simulink model "TSR_Scenario_005_cs"  (Simulink 7.6)
settings: Solver=ode5  SolverMode=SingleTasking  StartTime=0.0  StopTime=inf  FixedStep=1/100  RelTol=1e-3
PreLoadFcn:
  | check_NUTS_defined;
  | 
  | assignin('base',['model_closed_' bdroot],false);
  | 
  | addpath('../includes_TSR/includes_TSR');
  | 
  | switch version('-release')
  | case {'2010a', '2010b', '2011a'}
  | addpath('../includes_TSR/ML2010b');
  | otherwise
  | addpath('../includes_TSR/ML2011b');
  | end
InitFcn:
  | PreScanExperimentInitFcn(bdroot)
StopFcn:
  | PreScanExperimentStopFcn(bdroot)
undefined symbols (not defined in the file): DiscIntNeverNeededParam, InitImageOutputDir, x_ini_VRML, y_ini_VRML, z_ini_VRML
libraries: PreScanSimulinkLibrary x97, simulink x59, PreScanUsefulBlocks x33, simulink_extras x30, simulink_need_slupdate x1
(part 3 of 26)

== system TSR_Scenario_005_cs/FullCurtainSidedTrailer_1 ==
  SELF_FullCurtainSidedTrailer_1 : Inport  Tag=SELFInport
  Dynamics_Empty : Subsystem  Tag=Dynamics_Empty
      mask Display: port_label('output', 1, 'State');text(0,0,' Empty\n')
  SELF_Demux : Subsystem  Tag=DemuxSELF
      settings: DeleteFcn=PreScanBlockDeleteFcn;
      mask Type: PreScan Demux Block:
      mask Display:
      | port_label('output',1,'x [m]')
      | port_label('output',2,'y [m]')
      | port_label('output',3,'z [m]','texmode','on')
      | port_label('output',4,'Rotx [deg]','texmode','on')
      | port_label('output',5,'Roty [deg]','texmode','on')
      | port_label('output',6,'Rotz [deg]','texmode','on')
      | port_label('output',7,'GPS lat [deg]','texmode','on')
      | port_label('output',8,'GPS long [deg]','texmode','on')
      | port_label('output',9,'GPS alt [m]','texmode','on')
      | port_label('output',10,'Velocity [m/s]')
      | port_label('output',11,'Heading [deg]')
      | port_label('output',12,'Yaw rate [deg/s]')
  Terminator_{1..12} (12 alike) : Terminator
  STATE_FullCurtainSidedTrailer_1 : Outport  Tag=StateOutport
  wires:
  SELF_FullCurtainSidedTrailer_1/1 -> SELF_Demux/1(SELF)
  Dynamics_Empty/1(State) -> STATE_FullCurtainSidedTrailer_1/1
  SELF_Demux/1(x [m]) -> Terminator_1/1
  SELF_Demux/2(y [m]) -> Terminator_2/1
  SELF_Demux/3(z [m]) -> Terminator_3/1
  SELF_Demux/4(rotx [deg]) -> Terminator_4/1
  SELF_Demux/5(roty [deg]) -> Terminator_5/1
  SELF_Demux/6(rotz [deg]) -> Terminator_6/1
  SELF_Demux/7(GPS lat [deg]) -> Terminator_7/1
  SELF_Demux/8(GPS long [deg]) -> Terminator_8/1
  SELF_Demux/9(GPS alt [m]) -> Terminator_9/1
  SELF_Demux/10(velocity [m/s]) -> Terminator_10/1
  SELF_Demux/11(heading [deg]) -> Terminator_11/1
  SELF_Demux/12(yaw rate [deg/s]) -> Terminator_12/1

== system TSR_Scenario_005_cs/GetSensors ==
  dummy : Inport
  GetSensors : M-S-Function  FunctionName=GetSensorsBlock  Parameters=TimeStep NrOfSensors
  tag scope: SimCoreSensors=global
  wires:
  GetSensors/1 -> [SimCoreSensors]
  dummy/1 -> GetSensors/1

== system TSR_Scenario_005_cs/Honda_Pan_European_1 ==
  SELF_Honda_Pan_European_1 : Inport  Tag=SELFInport
  SELF_Demux : Subsystem  Tag=DemuxSELF
      settings: DeleteFcn=PreScanBlockDeleteFcn;
      mask Type: PreScan Demux Block:
      mask Display:
      | port_label('output',1,'x [m]')
      | port_label('output',2,'y [m]')
      | port_label('output',3,'z [m]','texmode','on')
      | port_label('output',4,'Rotx [deg]','texmode','on')
      | port_label('output',5,'Roty [deg]','texmode','on')
      | port_label('output',6,'Rotz [deg]','texmode','on')
      | port_label('output',7,'GPS lat [deg]','texmode','on')
      | port_label('output',8,'GPS long [deg]','texmode','on')
      | port_label('output',9,'GPS alt [m]','texmode','on')
      | port_label('output',10,'Velocity [m/s]')
      | port_label('output',11,'Heading [deg]')
      | port_label('output',12,'Yaw rate [deg/s]')
  Terminator_{1..12} (12 alike) : Terminator
  Trajectories : Subsystem  Tag=Trajectories
      settings: DeleteFcn=PreScanBlockDeleteFcn;
      mask Type: PreScan Source Block: Trajectories
      mask Description:
      | The Trajectory block contains the trajectories defined in the PreScan Experiment Editor. The output of the trajectory block is the (vehicle) State.
      | 
      | For more information, please click Help.
      mask Help: eval('openHelp(312)')
      mask Display: image(imread('Trajectory.png'));
  STATE_Honda_Pan_European_1 : Outport  Tag=StateOutport
  wires:
  SELF_Honda_Pan_European_1/1 -> SELF_Demux/1(SELF)
  Trajectories/1(State) -> STATE_Honda_Pan_European_1/1
  SELF_Demux/1(x [m]) -> Terminator_1/1
  SELF_Demux/2(y [m]) -> Terminator_2/1
  SELF_Demux/3(z [m]) -> Terminator_3/1
  SELF_Demux/4(rotx [deg]) -> Terminator_4/1
  SELF_Demux/5(roty [deg]) -> Terminator_5/1
  SELF_Demux/6(rotz [deg]) -> Terminator_6/1
  SELF_Demux/7(GPS lat [deg]) -> Terminator_7/1
  SELF_Demux/8(GPS long [deg]) -> Terminator_8/1
  SELF_Demux/9(GPS alt [m]) -> Terminator_9/1
  SELF_Demux/10(velocity [m/s]) -> Terminator_10/1
  SELF_Demux/11(heading [deg]) -> Terminator_11/1
  SELF_Demux/12(yaw rate [deg/s]) -> Terminator_12/1

== system TSR_Scenario_005_cs/Lightpost_1 ==
  note: Resources
      |
      | 1: LightMap\HeadLight LB and HB.png: HeadLight LB and HB
      | 2: LightMap\HeadLight LB.png: HeadLight LB
      | 3: LightMap\GeneralLight.png: GeneralLight
      | 4: LightMap\BrakeLight.png: BrakeLight
  note: Resources
  LightPostLight_Col : Constant  Tag=VisuLightConst  Value=[255 210 89]
  LightPostLight_ID : Constant  Tag=VisuLightConst  Value=[0]
  LightPostLight_Ori : Constant  Tag=VisuLightConst  Value=[0 0 0]
  LightPostLight_Pos : Constant  Tag=VisuLightConst  Value=[3.2599999904632568 -1.7702632248983718E-05 9.9388999938964844]
  LightPostLight_mscd : Constant  Tag=VisuLightConst  Value=[398.33]
  VISULightPostLight_Mux : Subsystem  Tag=MuxLights
      settings: DeleteFcn=PreScanBlockDeleteFcn;
      mask Type: PreScan Mux Block:
  VISULightPostLight_Lightpost_1 : Outport  Tag=VisuLightPostLightOutport  SampleTime=1/100
  wires:
  VISULightPostLight_Mux/1(Lights) -> VISULightPostLight_Lightpost_1/1
  LightPostLight_mscd/1 -> VISULightPostLight_Mux/1(MSCD [Cd])
  LightPostLight_Pos/1 -> VISULightPostLight_Mux/2(Position (xyz) [m])
  LightPostLight_Ori/1 -> VISULightPostLight_Mux/3(Orientation (RPY) [deg])
  LightPostLight_Col/1 -> VISULightPostLight_Mux/4(Color [-])
  LightPostLight_ID/1 -> VISULightPostLight_Mux/5(TextureResourceID [-])

== system TSR_Scenario_005_cs/Lightpost_10 ==

== system TSR_Scenario_005_cs/Lightpost_11 ==
  note: Resources
      |
      | 1: LightMap\HeadLight LB and HB.png: HeadLight LB and HB
      | 2: LightMap\HeadLight LB.png: HeadLight LB
      | 3: LightMap\GeneralLight.png: GeneralLight
      | 4: LightMap\BrakeLight.png: BrakeLight
  note: Resources
  LightPostLight_Col : Constant  Tag=VisuLightConst  Value=[255 210 89]
  LightPostLight_ID : Constant  Tag=VisuLightConst  Value=[0]
  LightPostLight_Ori : Constant  Tag=VisuLightConst  Value=[0 0 0]
  LightPostLight_Pos : Constant  Tag=VisuLightConst  Value=[3.2599999904632568 -1.7702632248983718E-05 9.9388999938964844]
  LightPostLight_mscd : Constant  Tag=VisuLightConst  Value=[398.33]
  VISULightPostLight_Mux : Subsystem  Tag=MuxLights
      settings: DeleteFcn=PreScanBlockDeleteFcn;
      mask Type: PreScan Mux Block:
  VISULightPostLight_Lightpost_11 : Outport  Tag=VisuLightPostLightOutport  SampleTime=1/100
  wires:
  VISULightPostLight_Mux/1(Lights) -> VISULightPostLight_Lightpost_11/1
  LightPostLight_mscd/1 -> VISULightPostLight_Mux/1(MSCD [Cd])
  LightPostLight_Pos/1 -> VISULightPostLight_Mux/2(Position (xyz) [m])
  LightPostLight_Ori/1 -> VISULightPostLight_Mux/3(Orientation (RPY) [deg])
  LightPostLight_Col/1 -> VISULightPostLight_Mux/4(Color [-])
  LightPostLight_ID/1 -> VISULightPostLight_Mux/5(TextureResourceID [-])

== system TSR_Scenario_005_cs/Lightpost_12 ==
  note: Resources
      |
      | 1: LightMap\HeadLight LB and HB.png: HeadLight LB and HB
      | 2: LightMap\HeadLight LB.png: HeadLight LB
      | 3: LightMap\GeneralLight.png: GeneralLight
      | 4: LightMap\BrakeLight.png: BrakeLight
  note: Resources
  LightPostLight_Col : Constant  Tag=VisuLightConst  Value=[255 210 89]
  LightPostLight_ID : Constant  Tag=VisuLightConst  Value=[0]
  LightPostLight_Ori : Constant  Tag=VisuLightConst  Value=[0 0 0]
  LightPostLight_Pos : Constant  Tag=VisuLightConst  Value=[3.2599999904632568 -1.7702632248983718E-05 9.9388999938964844]
  LightPostLight_mscd : Constant  Tag=VisuLightConst  Value=[398.33]
  VISULightPostLight_Mux : Subsystem  Tag=MuxLights
      settings: DeleteFcn=PreScanBlockDeleteFcn;
      mask Type: PreScan Mux Block:
  VISULightPostLight_Lightpost_12 : Outport  Tag=VisuLightPostLightOutport  SampleTime=1/100
  wires:
  VISULightPostLight_Mux/1(Lights) -> VISULightPostLight_Lightpost_12/1
  LightPostLight_mscd/1 -> VISULightPostLight_Mux/1(MSCD [Cd])
  LightPostLight_Pos/1 -> VISULightPostLight_Mux/2(Position (xyz) [m])
  LightPostLight_Ori/1 -> VISULightPostLight_Mux/3(Orientation (RPY) [deg])
  LightPostLight_Col/1 -> VISULightPostLight_Mux/4(Color [-])
  LightPostLight_ID/1 -> VISULightPostLight_Mux/5(TextureResourceID [-])

== system TSR_Scenario_005_cs/Lightpost_13 ==

== system TSR_Scenario_005_cs/Lightpost_14 ==

== system TSR_Scenario_005_cs/Lightpost_15 ==

== system TSR_Scenario_005_cs/Lightpost_16 ==

== system TSR_Scenario_005_cs/Lightpost_17 ==

== system TSR_Scenario_005_cs/Lightpost_18 ==

== system TSR_Scenario_005_cs/Lightpost_19 ==

== system TSR_Scenario_005_cs/Lightpost_2 ==
  note: Resources
      |
      | 1: LightMap\HeadLight LB and HB.png: HeadLight LB and HB
      | 2: LightMap\HeadLight LB.png: HeadLight LB
      | 3: LightMap\GeneralLight.png: GeneralLight
      | 4: LightMap\BrakeLight.png: BrakeLight
  note: Resources
  LightPostLight_Col : Constant  Tag=VisuLightConst  Value=[255 210 89]
  LightPostLight_ID : Constant  Tag=VisuLightConst  Value=[0]
  LightPostLight_Ori : Constant  Tag=VisuLightConst  Value=[0 0 0]
  LightPostLight_Pos : Constant  Tag=VisuLightConst  Value=[3.2599999904632568 -1.7702632248983718E-05 9.9388999938964844]
  LightPostLight_mscd : Constant  Tag=VisuLightConst  Value=[398.33]
  VISULightPostLight_Mux : Subsystem  Tag=MuxLights
      settings: DeleteFcn=PreScanBlockDeleteFcn;
      mask Type: PreScan Mux Block:
  VISULightPostLight_Lightpost_2 : Outport  Tag=VisuLightPostLightOutport  SampleTime=1/100
  wires:
  VISULightPostLight_Mux/1(Lights) -> VISULightPostLight_Lightpost_2/1
  LightPostLight_mscd/1 -> VISULightPostLight_Mux/1(MSCD [Cd])
  LightPostLight_Pos/1 -> VISULightPostLight_Mux/2(Position (xyz) [m])
  LightPostLight_Ori/1 -> VISULightPostLight_Mux/3(Orientation (RPY) [deg])
  LightPostLight_Col/1 -> VISULightPostLight_Mux/4(Color [-])
  LightPostLight_ID/1 -> VISULightPostLight_Mux/5(TextureResourceID [-])

== system TSR_Scenario_005_cs/Lightpost_20 ==

== system TSR_Scenario_005_cs/Lightpost_21 ==

== system TSR_Scenario_005_cs/Lightpost_22 ==

== system TSR_Scenario_005_cs/Lightpost_23 ==

== system TSR_Scenario_005_cs/Lightpost_24 ==

== system TSR_Scenario_005_cs/Lightpost_25 ==
  note: Resources
      |
      | 1: LightMap\HeadLight LB and HB.png: HeadLight LB and HB
      | 2: LightMap\HeadLight LB.png: HeadLight LB
      | 3: LightMap\GeneralLight.png: GeneralLight
      | 4: LightMap\BrakeLight.png: BrakeLight
  note: Resources
  LightPostLight_Col : Constant  Tag=VisuLightConst  Value=[255 210 89]
  LightPostLight_ID : Constant  Tag=VisuLightConst  Value=[0]
  LightPostLight_Ori : Constant  Tag=VisuLightConst  Value=[0 0 0]
  LightPostLight_Pos : Constant  Tag=VisuLightConst  Value=[3.2599999904632568 -1.7702632248983718E-05 9.9388999938964844]
  LightPostLight_mscd : Constant  Tag=VisuLightConst  Value=[398.33]
  VISULightPostLight_Mux : Subsystem  Tag=MuxLights
      settings: DeleteFcn=PreScanBlockDeleteFcn;
      mask Type: PreScan Mux Block:
  VISULightPostLight_Lightpost_25 : Outport  Tag=VisuLightPostLightOutport  SampleTime=1/100
  wires:
  VISULightPostLight_Mux/1(Lights) -> VISULightPostLight_Lightpost_25/1
  LightPostLight_mscd/1 -> VISULightPostLight_Mux/1(MSCD [Cd])
  LightPostLight_Pos/1 -> VISULightPostLight_Mux/2(Position (xyz) [m])
  LightPostLight_Ori/1 -> VISULightPostLight_Mux/3(Orientation (RPY) [deg])
  LightPostLight_Col/1 -> VISULightPostLight_Mux/4(Color [-])
  LightPostLight_ID/1 -> VISULightPostLight_Mux/5(TextureResourceID [-])

== system TSR_Scenario_005_cs/Lightpost_26 ==

== system TSR_Scenario_005_cs/Lightpost_27 ==
  note: Resources
      |
      | 1: LightMap\HeadLight LB and HB.png: HeadLight LB and HB
      | 2: LightMap\HeadLight LB.png: HeadLight LB
      | 3: LightMap\GeneralLight.png: GeneralLight
      | 4: LightMap\BrakeLight.png: BrakeLight
  note: Resources
  LightPostLight_Col : Constant  Tag=VisuLightConst  Value=[255 210 89]
  LightPostLight_ID : Constant  Tag=VisuLightConst  Value=[0]
  LightPostLight_Ori : Constant  Tag=VisuLightConst  Value=[0 0 0]
  LightPostLight_Pos : Constant  Tag=VisuLightConst  Value=[3.2599999904632568 -1.7702632248983718E-05 9.9388999938964844]
  LightPostLight_mscd : Constant  Tag=VisuLightConst  Value=[398.33]
  VISULightPostLight_Mux : Subsystem  Tag=MuxLights
      settings: DeleteFcn=PreScanBlockDeleteFcn;
      mask Type: PreScan Mux Block:
  VISULightPostLight_Lightpost_27 : Outport  Tag=VisuLightPostLightOutport  SampleTime=1/100
  wires:
  VISULightPostLight_Mux/1(Lights) -> VISULightPostLight_Lightpost_27/1
  LightPostLight_mscd/1 -> VISULightPostLight_Mux/1(MSCD [Cd])
  LightPostLight_Pos/1 -> VISULightPostLight_Mux/2(Position (xyz) [m])
  LightPostLight_Ori/1 -> VISULightPostLight_Mux/3(Orientation (RPY) [deg])
  LightPostLight_Col/1 -> VISULightPostLight_Mux/4(Color [-])
  LightPostLight_ID/1 -> VISULightPostLight_Mux/5(TextureResourceID [-])

== system TSR_Scenario_005_cs/Lightpost_28 ==

== system TSR_Scenario_005_cs/Lightpost_29 ==

== system TSR_Scenario_005_cs/Lightpost_3 ==
  note: Resources
      |
      | 1: LightMap\HeadLight LB and HB.png: HeadLight LB and HB
      | 2: LightMap\HeadLight LB.png: HeadLight LB
      | 3: LightMap\GeneralLight.png: GeneralLight
      | 4: LightMap\BrakeLight.png: BrakeLight
  note: Resources
  LightPostLight_Col : Constant  Tag=VisuLightConst  Value=[255 210 89]
  LightPostLight_ID : Constant  Tag=VisuLightConst  Value=[0]
  LightPostLight_Ori : Constant  Tag=VisuLightConst  Value=[0 0 0]
  LightPostLight_Pos : Constant  Tag=VisuLightConst  Value=[3.2599999904632568 -1.7702632248983718E-05 9.9388999938964844]
  LightPostLight_mscd : Constant  Tag=VisuLightConst  Value=[398.33]
  VISULightPostLight_Mux : Subsystem  Tag=MuxLights
      settings: DeleteFcn=PreScanBlockDeleteFcn;
      mask Type: PreScan Mux Block:
  VISULightPostLight_Lightpost_3 : Outport  Tag=VisuLightPostLightOutport  SampleTime=1/100
  wires:
  VISULightPostLight_Mux/1(Lights) -> VISULightPostLight_Lightpost_3/1
  LightPostLight_mscd/1 -> VISULightPostLight_Mux/1(MSCD [Cd])
  LightPostLight_Pos/1 -> VISULightPostLight_Mux/2(Position (xyz) [m])
  LightPostLight_Ori/1 -> VISULightPostLight_Mux/3(Orientation (RPY) [deg])
  LightPostLight_Col/1 -> VISULightPostLight_Mux/4(Color [-])
  LightPostLight_ID/1 -> VISULightPostLight_Mux/5(TextureResourceID [-])

== system TSR_Scenario_005_cs/Lightpost_30 ==

== system TSR_Scenario_005_cs/Lightpost_31 ==

== system TSR_Scenario_005_cs/Lightpost_32 ==

== system TSR_Scenario_005_cs/Lightpost_33 ==

== system TSR_Scenario_005_cs/Lightpost_34 ==
  note: Resources
      |
      | 1: LightMap\HeadLight LB and HB.png: HeadLight LB and HB
      | 2: LightMap\HeadLight LB.png: HeadLight LB
      | 3: LightMap\GeneralLight.png: GeneralLight
      | 4: LightMap\BrakeLight.png: BrakeLight
  note: Resources
  LightPostLight_Col : Constant  Tag=VisuLightConst  Value=[255 210 89]
  LightPostLight_ID : Constant  Tag=VisuLightConst  Value=[0]
  LightPostLight_Ori : Constant  Tag=VisuLightConst  Value=[0 0 0]
  LightPostLight_Pos : Constant  Tag=VisuLightConst  Value=[3.2599999904632568 -1.7702632248983718E-05 9.9388999938964844]
  LightPostLight_mscd : Constant  Tag=VisuLightConst  Value=[398.33]
  VISULightPostLight_Mux : Subsystem  Tag=MuxLights
      settings: DeleteFcn=PreScanBlockDeleteFcn;
      mask Type: PreScan Mux Block:
  VISULightPostLight_Lightpost_34 : Outport  Tag=VisuLightPostLightOutport  SampleTime=1/100
  wires:
  VISULightPostLight_Mux/1(Lights) -> VISULightPostLight_Lightpost_34/1
  LightPostLight_mscd/1 -> VISULightPostLight_Mux/1(MSCD [Cd])
  LightPostLight_Pos/1 -> VISULightPostLight_Mux/2(Position (xyz) [m])
  LightPostLight_Ori/1 -> VISULightPostLight_Mux/3(Orientation (RPY) [deg])
  LightPostLight_Col/1 -> VISULightPostLight_Mux/4(Color [-])
  LightPostLight_ID/1 -> VISULightPostLight_Mux/5(TextureResourceID [-])

== system TSR_Scenario_005_cs/Lightpost_35 ==

== system TSR_Scenario_005_cs/Lightpost_36 ==
  note: Resources
      |
      | 1: LightMap\HeadLight LB and HB.png: HeadLight LB and HB
      | 2: LightMap\HeadLight LB.png: HeadLight LB
      | 3: LightMap\GeneralLight.png: GeneralLight
      | 4: LightMap\BrakeLight.png: BrakeLight
  note: Resources
  LightPostLight_Col : Constant  Tag=VisuLightConst  Value=[255 210 89]
  LightPostLight_ID : Constant  Tag=VisuLightConst  Value=[0]
  LightPostLight_Ori : Constant  Tag=VisuLightConst  Value=[0 0 0]
  LightPostLight_Pos : Constant  Tag=VisuLightConst  Value=[3.2599999904632568 -1.7702632248983718E-05 9.9388999938964844]
  LightPostLight_mscd : Constant  Tag=VisuLightConst  Value=[398.33]
  VISULightPostLight_Mux : Subsystem  Tag=MuxLights
      settings: DeleteFcn=PreScanBlockDeleteFcn;
      mask Type: PreScan Mux Block:
  VISULightPostLight_Lightpost_36 : Outport  Tag=VisuLightPostLightOutport  SampleTime=1/100
  wires:
  VISULightPostLight_Mux/1(Lights) -> VISULightPostLight_Lightpost_36/1
  LightPostLight_mscd/1 -> VISULightPostLight_Mux/1(MSCD [Cd])
  LightPostLight_Pos/1 -> VISULightPostLight_Mux/2(Position (xyz) [m])
  LightPostLight_Ori/1 -> VISULightPostLight_Mux/3(Orientation (RPY) [deg])
  LightPostLight_Col/1 -> VISULightPostLight_Mux/4(Color [-])
  LightPostLight_ID/1 -> VISULightPostLight_Mux/5(TextureResourceID [-])

== system TSR_Scenario_005_cs/Lightpost_37 ==

== system TSR_Scenario_005_cs/Lightpost_38 ==
  note: Resources
      |
      | 1: LightMap\HeadLight LB and HB.png: HeadLight LB and HB
      | 2: LightMap\HeadLight LB.png: HeadLight LB
      | 3: LightMap\GeneralLight.png: GeneralLight
      | 4: LightMap\BrakeLight.png: BrakeLight
  note: Resources
  LightPostLight_Col : Constant  Tag=VisuLightConst  Value=[255 210 89]
  LightPostLight_ID : Constant  Tag=VisuLightConst  Value=[0]
  LightPostLight_Ori : Constant  Tag=VisuLightConst  Value=[0 0 0]
  LightPostLight_Pos : Constant  Tag=VisuLightConst  Value=[3.2599999904632568 -1.7702632248983718E-05 9.9388999938964844]
  LightPostLight_mscd : Constant  Tag=VisuLightConst  Value=[398.33]
  VISULightPostLight_Mux : Subsystem  Tag=MuxLights
      settings: DeleteFcn=PreScanBlockDeleteFcn;
      mask Type: PreScan Mux Block:
  VISULightPostLight_Lightpost_38 : Outport  Tag=VisuLightPostLightOutport  SampleTime=1/100
  wires:
  VISULightPostLight_Mux/1(Lights) -> VISULightPostLight_Lightpost_38/1
  LightPostLight_mscd/1 -> VISULightPostLight_Mux/1(MSCD [Cd])
  LightPostLight_Pos/1 -> VISULightPostLight_Mux/2(Position (xyz) [m])
  LightPostLight_Ori/1 -> VISULightPostLight_Mux/3(Orientation (RPY) [deg])
  LightPostLight_Col/1 -> VISULightPostLight_Mux/4(Color [-])
  LightPostLight_ID/1 -> VISULightPostLight_Mux/5(TextureResourceID [-])

== system TSR_Scenario_005_cs/Lightpost_39 ==

== system TSR_Scenario_005_cs/Lightpost_4 ==
  note: Resources
      |
      | 1: LightMap\HeadLight LB and HB.png: HeadLight LB and HB
      | 2: LightMap\HeadLight LB.png: HeadLight LB
      | 3: LightMap\GeneralLight.png: GeneralLight
      | 4: LightMap\BrakeLight.png: BrakeLight
  note: Resources
  LightPostLight_Col : Constant  Tag=VisuLightConst  Value=[255 210 89]
  LightPostLight_ID : Constant  Tag=VisuLightConst  Value=[0]
  LightPostLight_Ori : Constant  Tag=VisuLightConst  Value=[0 0 0]
  LightPostLight_Pos : Constant  Tag=VisuLightConst  Value=[3.2599999904632568 -1.7702632248983718E-05 9.9388999938964844]
  LightPostLight_mscd : Constant  Tag=VisuLightConst  Value=[398.33]
  VISULightPostLight_Mux : Subsystem  Tag=MuxLights
      settings: DeleteFcn=PreScanBlockDeleteFcn;
      mask Type: PreScan Mux Block:
  VISULightPostLight_Lightpost_4 : Outport  Tag=VisuLightPostLightOutport  SampleTime=1/100
  wires:
  VISULightPostLight_Mux/1(Lights) -> VISULightPostLight_Lightpost_4/1
  LightPostLight_mscd/1 -> VISULightPostLight_Mux/1(MSCD [Cd])
  LightPostLight_Pos/1 -> VISULightPostLight_Mux/2(Position (xyz) [m])
  LightPostLight_Ori/1 -> VISULightPostLight_Mux/3(Orientation (RPY) [deg])
  LightPostLight_Col/1 -> VISULightPostLight_Mux/4(Color [-])
  LightPostLight_ID/1 -> VISULightPostLight_Mux/5(TextureResourceID [-])

== system TSR_Scenario_005_cs/Lightpost_40 ==
  note: Resources
      |
      | 1: LightMap\HeadLight LB and HB.png: HeadLight LB and HB
      | 2: LightMap\HeadLight LB.png: HeadLight LB
      | 3: LightMap\GeneralLight.png: GeneralLight
      | 4: LightMap\BrakeLight.png: BrakeLight
  note: Resources
  LightPostLight_Col : Constant  Tag=VisuLightConst  Value=[255 210 89]
  LightPostLight_ID : Constant  Tag=VisuLightConst  Value=[0]
  LightPostLight_Ori : Constant  Tag=VisuLightConst  Value=[0 0 0]
  LightPostLight_Pos : Constant  Tag=VisuLightConst  Value=[3.2599999904632568 -1.7702632248983718E-05 9.9388999938964844]
  LightPostLight_mscd : Constant  Tag=VisuLightConst  Value=[398.33]
  VISULightPostLight_Mux : Subsystem  Tag=MuxLights
      settings: DeleteFcn=PreScanBlockDeleteFcn;
      mask Type: PreScan Mux Block:
  VISULightPostLight_Lightpost_40 : Outport  Tag=VisuLightPostLightOutport  SampleTime=1/100
  wires:
  VISULightPostLight_Mux/1(Lights) -> VISULightPostLight_Lightpost_40/1
  LightPostLight_mscd/1 -> VISULightPostLight_Mux/1(MSCD [Cd])
  LightPostLight_Pos/1 -> VISULightPostLight_Mux/2(Position (xyz) [m])
  LightPostLight_Ori/1 -> VISULightPostLight_Mux/3(Orientation (RPY) [deg])
  LightPostLight_Col/1 -> VISULightPostLight_Mux/4(Color [-])
  LightPostLight_ID/1 -> VISULightPostLight_Mux/5(TextureResourceID [-])

== system TSR_Scenario_005_cs/Lightpost_41 ==

== system TSR_Scenario_005_cs/Lightpost_42 ==
  note: Resources
      |
      | 1: LightMap\HeadLight LB and HB.png: HeadLight LB and HB
      | 2: LightMap\HeadLight LB.png: HeadLight LB
      | 3: LightMap\GeneralLight.png: GeneralLight
      | 4: LightMap\BrakeLight.png: BrakeLight
  note: Resources
  LightPostLight_Col : Constant  Tag=VisuLightConst  Value=[255 210 89]
  LightPostLight_ID : Constant  Tag=VisuLightConst  Value=[0]
  LightPostLight_Ori : Constant  Tag=VisuLightConst  Value=[0 0 0]
  LightPostLight_Pos : Constant  Tag=VisuLightConst  Value=[3.2599999904632568 -1.7702632248983718E-05 9.9388999938964844]
  LightPostLight_mscd : Constant  Tag=VisuLightConst  Value=[398.33]
  VISULightPostLight_Mux : Subsystem  Tag=MuxLights
      settings: DeleteFcn=PreScanBlockDeleteFcn;
      mask Type: PreScan Mux Block:
  VISULightPostLight_Lightpost_42 : Outport  Tag=VisuLightPostLightOutport  SampleTime=1/100
  wires:
  VISULightPostLight_Mux/1(Lights) -> VISULightPostLight_Lightpost_42/1
  LightPostLight_mscd/1 -> VISULightPostLight_Mux/1(MSCD [Cd])
  LightPostLight_Pos/1 -> VISULightPostLight_Mux/2(Position (xyz) [m])
  LightPostLight_Ori/1 -> VISULightPostLight_Mux/3(Orientation (RPY) [deg])
  LightPostLight_Col/1 -> VISULightPostLight_Mux/4(Color [-])
  LightPostLight_ID/1 -> VISULightPostLight_Mux/5(TextureResourceID [-])

== system TSR_Scenario_005_cs/Lightpost_43 ==

== system TSR_Scenario_005_cs/Lightpost_44 ==
  note: Resources
      |
      | 1: LightMap\HeadLight LB and HB.png: HeadLight LB and HB
      | 2: LightMap\HeadLight LB.png: HeadLight LB
      | 3: LightMap\GeneralLight.png: GeneralLight
      | 4: LightMap\BrakeLight.png: BrakeLight
  note: Resources
  LightPostLight_Col : Constant  Tag=VisuLightConst  Value=[255 210 89]
  LightPostLight_ID : Constant  Tag=VisuLightConst  Value=[0]
  LightPostLight_Ori : Constant  Tag=VisuLightConst  Value=[0 0 0]
  LightPostLight_Pos : Constant  Tag=VisuLightConst  Value=[3.2599999904632568 -1.7702632248983718E-05 9.9388999938964844]
  LightPostLight_mscd : Constant  Tag=VisuLightConst  Value=[398.33]
  VISULightPostLight_Mux : Subsystem  Tag=MuxLights
      settings: DeleteFcn=PreScanBlockDeleteFcn;
      mask Type: PreScan Mux Block:
  VISULightPostLight_Lightpost_44 : Outport  Tag=VisuLightPostLightOutport  SampleTime=1/100
  wires:
  VISULightPostLight_Mux/1(Lights) -> VISULightPostLight_Lightpost_44/1
  LightPostLight_mscd/1 -> VISULightPostLight_Mux/1(MSCD [Cd])
  LightPostLight_Pos/1 -> VISULightPostLight_Mux/2(Position (xyz) [m])
  LightPostLight_Ori/1 -> VISULightPostLight_Mux/3(Orientation (RPY) [deg])
  LightPostLight_Col/1 -> VISULightPostLight_Mux/4(Color [-])
  LightPostLight_ID/1 -> VISULightPostLight_Mux/5(TextureResourceID [-])

== system TSR_Scenario_005_cs/Lightpost_45 ==

== system TSR_Scenario_005_cs/Lightpost_46 ==

== system TSR_Scenario_005_cs/Lightpost_47 ==

== system TSR_Scenario_005_cs/Lightpost_48 ==

== system TSR_Scenario_005_cs/Lightpost_49 ==

== system TSR_Scenario_005_cs/Lightpost_5 ==
  note: Resources
      |
      | 1: LightMap\HeadLight LB and HB.png: HeadLight LB and HB
      | 2: LightMap\HeadLight LB.png: HeadLight LB
      | 3: LightMap\GeneralLight.png: GeneralLight
      | 4: LightMap\BrakeLight.png: BrakeLight
  note: Resources
  LightPostLight_Col : Constant  Tag=VisuLightConst  Value=[255 210 89]
  LightPostLight_ID : Constant  Tag=VisuLightConst  Value=[0]
  LightPostLight_Ori : Constant  Tag=VisuLightConst  Value=[0 0 0]
  LightPostLight_Pos : Constant  Tag=VisuLightConst  Value=[3.2599999904632568 -1.7702632248983718E-05 9.9388999938964844]
  LightPostLight_mscd : Constant  Tag=VisuLightConst  Value=[398.33]
  VISULightPostLight_Mux : Subsystem  Tag=MuxLights
      settings: DeleteFcn=PreScanBlockDeleteFcn;
      mask Type: PreScan Mux Block:
  VISULightPostLight_Lightpost_5 : Outport  Tag=VisuLightPostLightOutport  SampleTime=1/100
  wires:
  VISULightPostLight_Mux/1(Lights) -> VISULightPostLight_Lightpost_5/1
  LightPostLight_mscd/1 -> VISULightPostLight_Mux/1(MSCD [Cd])
  LightPostLight_Pos/1 -> VISULightPostLight_Mux/2(Position (xyz) [m])
  LightPostLight_Ori/1 -> VISULightPostLight_Mux/3(Orientation (RPY) [deg])
  LightPostLight_Col/1 -> VISULightPostLight_Mux/4(Color [-])
  LightPostLight_ID/1 -> VISULightPostLight_Mux/5(TextureResourceID [-])

== system TSR_Scenario_005_cs/Lightpost_50 ==

== system TSR_Scenario_005_cs/Lightpost_51 ==

== system TSR_Scenario_005_cs/Lightpost_52 ==

== system TSR_Scenario_005_cs/Lightpost_53 ==

== system TSR_Scenario_005_cs/Lightpost_54 ==
  note: Resources
      |
      | 1: LightMap\HeadLight LB and HB.png: HeadLight LB and HB
      | 2: LightMap\HeadLight LB.png: HeadLight LB
      | 3: LightMap\GeneralLight.png: GeneralLight
      | 4: LightMap\BrakeLight.png: BrakeLight
  note: Resources
  LightPostLight_Col : Constant  Tag=VisuLightConst  Value=[255 210 89]
  LightPostLight_ID : Constant  Tag=VisuLightConst  Value=[0]
  LightPostLight_Ori : Constant  Tag=VisuLightConst  Value=[0 0 0]
  LightPostLight_Pos : Constant  Tag=VisuLightConst  Value=[3.2599999904632568 -1.7702632248983718E-05 9.9388999938964844]
  LightPostLight_mscd : Constant  Tag=VisuLightConst  Value=[398.33]
  VISULightPostLight_Mux : Subsystem  Tag=MuxLights
      settings: DeleteFcn=PreScanBlockDeleteFcn;
      mask Type: PreScan Mux Block:
  VISULightPostLight_Lightpost_54 : Outport  Tag=VisuLightPostLightOutport  SampleTime=1/100
  wires:
  VISULightPostLight_Mux/1(Lights) -> VISULightPostLight_Lightpost_54/1
  LightPostLight_mscd/1 -> VISULightPostLight_Mux/1(MSCD [Cd])
  LightPostLight_Pos/1 -> VISULightPostLight_Mux/2(Position (xyz) [m])
  LightPostLight_Ori/1 -> VISULightPostLight_Mux/3(Orientation (RPY) [deg])
  LightPostLight_Col/1 -> VISULightPostLight_Mux/4(Color [-])
  LightPostLight_ID/1 -> VISULightPostLight_Mux/5(TextureResourceID [-])

== system TSR_Scenario_005_cs/Lightpost_6 ==

== system TSR_Scenario_005_cs/Lightpost_7 ==

== system TSR_Scenario_005_cs/Lightpost_8 ==

== system TSR_Scenario_005_cs/Lightpost_9 ==

== system TSR_Scenario_005_cs/Male_RegularAni_1 ==
  SELF_Male_RegularAni_1 : Inport  Tag=SELFInport
  SELF_Demux : Subsystem  Tag=DemuxSELF
      settings: DeleteFcn=PreScanBlockDeleteFcn;
      mask Type: PreScan Demux Block:
      mask Display:
      | port_label('output',1,'x [m]')
      | port_label('output',2,'y [m]')
      | port_label('output',3,'z [m]','texmode','on')
      | port_label('output',4,'Rotx [deg]','texmode','on')
      | port_label('output',5,'Roty [deg]','texmode','on')
      | port_label('output',6,'Rotz [deg]','texmode','on')
      | port_label('output',7,'GPS lat [deg]','texmode','on')
      | port_label('output',8,'GPS long [deg]','texmode','on')
      | port_label('output',9,'GPS alt [m]','texmode','on')
      | port_label('output',10,'Velocity [m/s]')
      | port_label('output',11,'Heading [deg]')
      | port_label('output',12,'Yaw rate [deg/s]')
  Terminator_{1..12} (12 alike) : Terminator
  Trajectories : Subsystem  Tag=Trajectories
      settings: DeleteFcn=PreScanBlockDeleteFcn;
      mask Type: PreScan Source Block: Trajectories
      mask Description:
      | The Trajectory block contains the trajectories defined in the PreScan Experiment Editor. The output of the trajectory block is the (vehicle) State.
      | 
      | For more information, please click Help.
      mask Help: eval('openHelp(312)')
      mask Display: image(imread('Trajectory.png'));
  STATE_Male_RegularAni_1 : Outport  Tag=StateOutport
  wires:
  SELF_Male_RegularAni_1/1 -> SELF_Demux/1(SELF)
  Trajectories/1(State) -> STATE_Male_RegularAni_1/1
  SELF_Demux/1(x [m]) -> Terminator_1/1
  SELF_Demux/2(y [m]) -> Terminator_2/1
  SELF_Demux/3(z [m]) -> Terminator_3/1
  SELF_Demux/4(rotx [deg]) -> Terminator_4/1
  SELF_Demux/5(roty [deg]) -> Terminator_5/1
  SELF_Demux/6(rotz [deg]) -> Terminator_6/1
  SELF_Demux/7(GPS lat [deg]) -> Terminator_7/1
  SELF_Demux/8(GPS long [deg]) -> Terminator_8/1
  SELF_Demux/9(GPS alt [m]) -> Terminator_9/1
  SELF_Demux/10(velocity [m/s]) -> Terminator_10/1
  SELF_Demux/11(heading [deg]) -> Terminator_11/1
  SELF_Demux/12(yaw rate [deg/s]) -> Terminator_12/1

== system TSR_Scenario_005_cs/Male_Regular_wBackpack_1 ==
  SELF_Male_Regular_wBackpack_1 : Inport  Tag=SELFInport
  SELF_Demux : Subsystem  Tag=DemuxSELF
      settings: DeleteFcn=PreScanBlockDeleteFcn;
      mask Type: PreScan Demux Block:
      mask Display:
      | port_label('output',1,'x [m]')
      | port_label('output',2,'y [m]')
      | port_label('output',3,'z [m]','texmode','on')
      | port_label('output',4,'Rotx [deg]','texmode','on')
      | port_label('output',5,'Roty [deg]','texmode','on')
      | port_label('output',6,'Rotz [deg]','texmode','on')
      | port_label('output',7,'GPS lat [deg]','texmode','on')
      | port_label('output',8,'GPS long [deg]','texmode','on')
      | port_label('output',9,'GPS alt [m]','texmode','on')
      | port_label('output',10,'Velocity [m/s]')
      | port_label('output',11,'Heading [deg]')
      | port_label('output',12,'Yaw rate [deg/s]')
  Terminator_{1..12} (12 alike) : Terminator
  Trajectories : Subsystem  Tag=Trajectories
      settings: DeleteFcn=PreScanBlockDeleteFcn;
      mask Type: PreScan Source Block: Trajectories
      mask Description:
      | The Trajectory block contains the trajectories defined in the PreScan Experiment Editor. The output of the trajectory block is the (vehicle) State.
      | 
      | For more information, please click Help.
      mask Help: eval('openHelp(312)')
      mask Display: image(imread('Trajectory.png'));
  STATE_Male_Regular_wBackpack_1 : Outport  Tag=StateOutport
  wires:
  SELF_Male_Regular_wBackpack_1/1 -> SELF_Demux/1(SELF)
  Trajectories/1(State) -> STATE_Male_Regular_wBackpack_1/1
  SELF_Demux/1(x [m]) -> Terminator_1/1
  SELF_Demux/2(y [m]) -> Terminator_2/1
  SELF_Demux/3(z [m]) -> Terminator_3/1
  SELF_Demux/4(rotx [deg]) -> Terminator_4/1
  SELF_Demux/5(roty [deg]) -> Terminator_5/1
  SELF_Demux/6(rotz [deg]) -> Terminator_6/1
  SELF_Demux/7(GPS lat [deg]) -> Terminator_7/1
  SELF_Demux/8(GPS long [deg]) -> Terminator_8/1
  SELF_Demux/9(GPS alt [m]) -> Terminator_9/1
  SELF_Demux/10(velocity [m/s]) -> Terminator_10/1
  SELF_Demux/11(heading [deg]) -> Terminator_11/1
  SELF_Demux/12(yaw rate [deg/s]) -> Terminator_12/1

== system TSR_Scenario_005_cs/Male_Regular_wBicycle_1 ==
  SELF_Male_Regular_wBicycle_1 : Inport  Tag=SELFInport
  SELF_Demux : Subsystem  Tag=DemuxSELF
      settings: DeleteFcn=PreScanBlockDeleteFcn;
      mask Type: PreScan Demux Block:
      mask Display:
      | port_label('output',1,'x [m]')
      | port_label('output',2,'y [m]')
      | port_label('output',3,'z [m]','texmode','on')
      | port_label('output',4,'Rotx [deg]','texmode','on')
      | port_label('output',5,'Roty [deg]','texmode','on')
      | port_label('output',6,'Rotz [deg]','texmode','on')
      | port_label('output',7,'GPS lat [deg]','texmode','on')
      | port_label('output',8,'GPS long [deg]','texmode','on')
      | port_label('output',9,'GPS alt [m]','texmode','on')
      | port_label('output',10,'Velocity [m/s]')
      | port_label('output',11,'Heading [deg]')
      | port_label('output',12,'Yaw rate [deg/s]')
  Terminator_{1..12} (12 alike) : Terminator
  Trajectories : Subsystem  Tag=Trajectories
      settings: DeleteFcn=PreScanBlockDeleteFcn;
      mask Type: PreScan Source Block: Trajectories
      mask Description:
      | The Trajectory block contains the trajectories defined in the PreScan Experiment Editor. The output of the trajectory block is the (vehicle) State.
      | 
      | For more information, please click Help.
      mask Help: eval('openHelp(312)')
      mask Display: image(imread('Trajectory.png'));
  STATE_Male_Regular_wBicycle_1 : Outport  Tag=StateOutport
  wires:
  SELF_Male_Regular_wBicycle_1/1 -> SELF_Demux/1(SELF)
  Trajectories/1(State) -> STATE_Male_Regular_wBicycle_1/1
  SELF_Demux/1(x [m]) -> Terminator_1/1
  SELF_Demux/2(y [m]) -> Terminator_2/1
  SELF_Demux/3(z [m]) -> Terminator_3/1
  SELF_Demux/4(rotx [deg]) -> Terminator_4/1
  SELF_Demux/5(roty [deg]) -> Terminator_5/1
  SELF_Demux/6(rotz [deg]) -> Terminator_6/1
  SELF_Demux/7(GPS lat [deg]) -> Terminator_7/1
  SELF_Demux/8(GPS long [deg]) -> Terminator_8/1
  SELF_Demux/9(GPS alt [m]) -> Terminator_9/1
  SELF_Demux/10(velocity [m/s]) -> Terminator_10/1
  SELF_Demux/11(heading [deg]) -> Terminator_11/1
  SELF_Demux/12(yaw rate [deg/s]) -> Terminator_12/1

== system TSR_Scenario_005_cs/Male_Regular_wSuitcase_1 ==
  SELF_Male_Regular_wSuitcase_1 : Inport  Tag=SELFInport
  SELF_Demux : Subsystem  Tag=DemuxSELF
      settings: DeleteFcn=PreScanBlockDeleteFcn;
      mask Type: PreScan Demux Block:
      mask Display:
      | port_label('output',1,'x [m]')
      | port_label('output',2,'y [m]')
      | port_label('output',3,'z [m]','texmode','on')
      | port_label('output',4,'Rotx [deg]','texmode','on')
      | port_label('output',5,'Roty [deg]','texmode','on')
      | port_label('output',6,'Rotz [deg]','texmode','on')
      | port_label('output',7,'GPS lat [deg]','texmode','on')
      | port_label('output',8,'GPS long [deg]','texmode','on')
      | port_label('output',9,'GPS alt [m]','texmode','on')
      | port_label('output',10,'Velocity [m/s]')
      | port_label('output',11,'Heading [deg]')
      | port_label('output',12,'Yaw rate [deg/s]')
  Terminator_{1..12} (12 alike) : Terminator
  Trajectories : Subsystem  Tag=Trajectories
      settings: DeleteFcn=PreScanBlockDeleteFcn;
      mask Type: PreScan Source Block: Trajectories
      mask Description:
      | The Trajectory block contains the trajectories defined in the PreScan Experiment Editor. The output of the trajectory block is the (vehicle) State.
      | 
      | For more information, please click Help.
      mask Help: eval('openHelp(312)')
      mask Display: image(imread('Trajectory.png'));
  STATE_Male_Regular_wSuitcase_1 : Outport  Tag=StateOutport
  wires:
  SELF_Male_Regular_wSuitcase_1/1 -> SELF_Demux/1(SELF)
  Trajectories/1(State) -> STATE_Male_Regular_wSuitcase_1/1
  SELF_Demux/1(x [m]) -> Terminator_1/1
  SELF_Demux/2(y [m]) -> Terminator_2/1
  SELF_Demux/3(z [m]) -> Terminator_3/1
  SELF_Demux/4(rotx [deg]) -> Terminator_4/1
  SELF_Demux/5(roty [deg]) -> Terminator_5/1
  SELF_Demux/6(rotz [deg]) -> Terminator_6/1
  SELF_Demux/7(GPS lat [deg]) -> Terminator_7/1
  SELF_Demux/8(GPS long [deg]) -> Terminator_8/1
  SELF_Demux/9(GPS alt [m]) -> Terminator_9/1
  SELF_Demux/10(velocity [m/s]) -> Terminator_10/1
  SELF_Demux/11(heading [deg]) -> Terminator_11/1
  SELF_Demux/12(yaw rate [deg/s]) -> Terminator_12/1

== system TSR_Scenario_005_cs/Mazda_RX8_1 ==
  SELF_Mazda_RX8_1 : Inport  Tag=SELFInport
  SELF_Demux : Subsystem  Tag=DemuxSELF
      settings: DeleteFcn=PreScanBlockDeleteFcn;
      mask Type: PreScan Demux Block:
      mask Display:
      | port_label('output',1,'x [m]')
      | port_label('output',2,'y [m]')
      | port_label('output',3,'z [m]','texmode','on')
      | port_label('output',4,'Rotx [deg]','texmode','on')
      | port_label('output',5,'Roty [deg]','texmode','on')
      | port_label('output',6,'Rotz [deg]','texmode','on')
      | port_label('output',7,'GPS lat [deg]','texmode','on')
      | port_label('output',8,'GPS long [deg]','texmode','on')
      | port_label('output',9,'GPS alt [m]','texmode','on')
      | port_label('output',10,'Velocity [m/s]')
      | port_label('output',11,'Heading [deg]')
      | port_label('output',12,'Yaw rate [deg/s]')
  Terminator_{1..12} (12 alike) : Terminator
  Trajectories : Subsystem  Tag=Trajectories
      settings: DeleteFcn=PreScanBlockDeleteFcn;
      mask Type: PreScan Source Block: Trajectories
      mask Description:
      | The Trajectory block contains the trajectories defined in the PreScan Experiment Editor. The output of the trajectory block is the (vehicle) State.
      | 
      | For more information, please click Help.
      mask Help: eval('openHelp(312)')
      mask Display: image(imread('Trajectory.png'));
  STATE_Mazda_RX8_1 : Outport  Tag=StateOutport
  wires:
  SELF_Mazda_RX8_1/1 -> SELF_Demux/1(SELF)
  Trajectories/1(State) -> STATE_Mazda_RX8_1/1
  SELF_Demux/1(x [m]) -> Terminator_1/1
  SELF_Demux/2(y [m]) -> Terminator_2/1
  SELF_Demux/3(z [m]) -> Terminator_3/1
  SELF_Demux/4(rotx [deg]) -> Terminator_4/1
  SELF_Demux/5(roty [deg]) -> Terminator_5/1
  SELF_Demux/6(rotz [deg]) -> Terminator_6/1
  SELF_Demux/7(GPS lat [deg]) -> Terminator_7/1
  SELF_Demux/8(GPS long [deg]) -> Terminator_8/1
  SELF_Demux/9(GPS alt [m]) -> Terminator_9/1
  SELF_Demux/10(velocity [m/s]) -> Terminator_10/1
  SELF_Demux/11(heading [deg]) -> Terminator_11/1
  SELF_Demux/12(yaw rate [deg/s]) -> Terminator_12/1

== system TSR_Scenario_005_cs/Mazda_RX8_2 ==
  SELF_Mazda_RX8_2 : Inport  Tag=SELFInport
  Dynamics_Empty : Subsystem  Tag=Dynamics_Empty
      mask Display: port_label('output', 1, 'State');text(0,0,' Empty\n')
  SELF_Demux : Subsystem  Tag=DemuxSELF
      settings: DeleteFcn=PreScanBlockDeleteFcn;
      mask Type: PreScan Demux Block:
      mask Display:
      | port_label('output',1,'x [m]')
      | port_label('output',2,'y [m]')
      | port_label('output',3,'z [m]','texmode','on')
      | port_label('output',4,'Rotx [deg]','texmode','on')
      | port_label('output',5,'Roty [deg]','texmode','on')
      | port_label('output',6,'Rotz [deg]','texmode','on')
      | port_label('output',7,'GPS lat [deg]','texmode','on')
      | port_label('output',8,'GPS long [deg]','texmode','on')
      | port_label('output',9,'GPS alt [m]','texmode','on')
      | port_label('output',10,'Velocity [m/s]')
      | port_label('output',11,'Heading [deg]')
      | port_label('output',12,'Yaw rate [deg/s]')
  Terminator_{1..12} (12 alike) : Terminator
  STATE_Mazda_RX8_2 : Outport  Tag=StateOutport
  wires:
  SELF_Mazda_RX8_2/1 -> SELF_Demux/1(SELF)
  Dynamics_Empty/1(State) -> STATE_Mazda_RX8_2/1
  SELF_Demux/1(x [m]) -> Terminator_1/1
  SELF_Demux/2(y [m]) -> Terminator_2/1
  SELF_Demux/3(z [m]) -> Terminator_3/1
  SELF_Demux/4(rotx [deg]) -> Terminator_4/1
  SELF_Demux/5(roty [deg]) -> Terminator_5/1
  SELF_Demux/6(rotz [deg]) -> Terminator_6/1
  SELF_Demux/7(GPS lat [deg]) -> Terminator_7/1
  SELF_Demux/8(GPS long [deg]) -> Terminator_8/1
  SELF_Demux/9(GPS alt [m]) -> Terminator_9/1
  SELF_Demux/10(velocity [m/s]) -> Terminator_10/1
  SELF_Demux/11(heading [deg]) -> Terminator_11/1
  SELF_Demux/12(yaw rate [deg/s]) -> Terminator_12/1

== system TSR_Scenario_005_cs/Mazda_RX8_3 ==
  SELF_Mazda_RX8_3 : Inport  Tag=SELFInport
  Dynamics_Empty : Subsystem  Tag=Dynamics_Empty
      mask Display: port_label('output', 1, 'State');text(0,0,' Empty\n')
  SELF_Demux : Subsystem  Tag=DemuxSELF
      settings: DeleteFcn=PreScanBlockDeleteFcn;
      mask Type: PreScan Demux Block:
      mask Display:
      | port_label('output',1,'x [m]')
      | port_label('output',2,'y [m]')
      | port_label('output',3,'z [m]','texmode','on')
      | port_label('output',4,'Rotx [deg]','texmode','on')
      | port_label('output',5,'Roty [deg]','texmode','on')
      | port_label('output',6,'Rotz [deg]','texmode','on')
      | port_label('output',7,'GPS lat [deg]','texmode','on')
      | port_label('output',8,'GPS long [deg]','texmode','on')
      | port_label('output',9,'GPS alt [m]','texmode','on')
      | port_label('output',10,'Velocity [m/s]')
      | port_label('output',11,'Heading [deg]')
      | port_label('output',12,'Yaw rate [deg/s]')
  Terminator_{1..12} (12 alike) : Terminator
  STATE_Mazda_RX8_3 : Outport  Tag=StateOutport
  wires:
  SELF_Mazda_RX8_3/1 -> SELF_Demux/1(SELF)
  Dynamics_Empty/1(State) -> STATE_Mazda_RX8_3/1
  SELF_Demux/1(x [m]) -> Terminator_1/1
  SELF_Demux/2(y [m]) -> Terminator_2/1
  SELF_Demux/3(z [m]) -> Terminator_3/1
  SELF_Demux/4(rotx [deg]) -> Terminator_4/1
  SELF_Demux/5(roty [deg]) -> Terminator_5/1
  SELF_Demux/6(rotz [deg]) -> Terminator_6/1
  SELF_Demux/7(GPS lat [deg]) -> Terminator_7/1
  SELF_Demux/8(GPS long [deg]) -> Terminator_8/1
  SELF_Demux/9(GPS alt [m]) -> Terminator_9/1
  SELF_Demux/10(velocity [m/s]) -> Terminator_10/1
  SELF_Demux/11(heading [deg]) -> Terminator_11/1
  SELF_Demux/12(yaw rate [deg/s]) -> Terminator_12/1

== system TSR_Scenario_005_cs/Nissan_Cabstar_1 ==
  SELF_Nissan_Cabstar_1 : Inport  Tag=SELFInport
  Dynamics_Empty : Subsystem  Tag=Dynamics_Empty
      mask Display: port_label('output', 1, 'State');text(0,0,' Empty\n')
  SELF_Demux : Subsystem  Tag=DemuxSELF
      settings: DeleteFcn=PreScanBlockDeleteFcn;
      mask Type: PreScan Demux Block:
      mask Display:
      | port_label('output',1,'x [m]')
      | port_label('output',2,'y [m]')
      | port_label('output',3,'z [m]','texmode','on')
      | port_label('output',4,'Rotx [deg]','texmode','on')
      | port_label('output',5,'Roty [deg]','texmode','on')
      | port_label('output',6,'Rotz [deg]','texmode','on')
      | port_label('output',7,'GPS lat [deg]','texmode','on')
      | port_label('output',8,'GPS long [deg]','texmode','on')
      | port_label('output',9,'GPS alt [m]','texmode','on')
      | port_label('output',10,'Velocity [m/s]')
      | port_label('output',11,'Heading [deg]')
      | port_label('output',12,'Yaw rate [deg/s]')
  Terminator_{1..12} (12 alike) : Terminator
  STATE_Nissan_Cabstar_1 : Outport  Tag=StateOutport
  wires:
  SELF_Nissan_Cabstar_1/1 -> SELF_Demux/1(SELF)
  Dynamics_Empty/1(State) -> STATE_Nissan_Cabstar_1/1
  SELF_Demux/1(x [m]) -> Terminator_1/1
  SELF_Demux/2(y [m]) -> Terminator_2/1
  SELF_Demux/3(z [m]) -> Terminator_3/1
  SELF_Demux/4(rotx [deg]) -> Terminator_4/1
  SELF_Demux/5(roty [deg]) -> Terminator_5/1
  SELF_Demux/6(rotz [deg]) -> Terminator_6/1
  SELF_Demux/7(GPS lat [deg]) -> Terminator_7/1
  SELF_Demux/8(GPS long [deg]) -> Terminator_8/1
  SELF_Demux/9(GPS alt [m]) -> Terminator_9/1
  SELF_Demux/10(velocity [m/s]) -> Terminator_10/1
  SELF_Demux/11(heading [deg]) -> Terminator_11/1
  SELF_Demux/12(yaw rate [deg/s]) -> Terminator_12/1

== system TSR_Scenario_005_cs/Nissan_Cabstar_2 ==
  SELF_Nissan_Cabstar_2 : Inport  Tag=SELFInport
  Dynamics_Empty : Subsystem  Tag=Dynamics_Empty
      mask Display: port_label('output', 1, 'State');text(0,0,' Empty\n')
  SELF_Demux : Subsystem  Tag=DemuxSELF
      settings: DeleteFcn=PreScanBlockDeleteFcn;
      mask Type: PreScan Demux Block:
      mask Display:
      | port_label('output',1,'x [m]')
      | port_label('output',2,'y [m]')
      | port_label('output',3,'z [m]','texmode','on')
      | port_label('output',4,'Rotx [deg]','texmode','on')
      | port_label('output',5,'Roty [deg]','texmode','on')
      | port_label('output',6,'Rotz [deg]','texmode','on')
      | port_label('output',7,'GPS lat [deg]','texmode','on')
      | port_label('output',8,'GPS long [deg]','texmode','on')
      | port_label('output',9,'GPS alt [m]','texmode','on')
      | port_label('output',10,'Velocity [m/s]')
      | port_label('output',11,'Heading [deg]')
      | port_label('output',12,'Yaw rate [deg/s]')
  Terminator_{1..12} (12 alike) : Terminator
  STATE_Nissan_Cabstar_2 : Outport  Tag=StateOutport
  wires:
  SELF_Nissan_Cabstar_2/1 -> SELF_Demux/1(SELF)
  Dynamics_Empty/1(State) -> STATE_Nissan_Cabstar_2/1
  SELF_Demux/1(x [m]) -> Terminator_1/1
  SELF_Demux/2(y [m]) -> Terminator_2/1
  SELF_Demux/3(z [m]) -> Terminator_3/1
  SELF_Demux/4(rotx [deg]) -> Terminator_4/1
  SELF_Demux/5(roty [deg]) -> Terminator_5/1
  SELF_Demux/6(rotz [deg]) -> Terminator_6/1
  SELF_Demux/7(GPS lat [deg]) -> Terminator_7/1
  SELF_Demux/8(GPS long [deg]) -> Terminator_8/1
  SELF_Demux/9(GPS alt [m]) -> Terminator_9/1
  SELF_Demux/10(velocity [m/s]) -> Terminator_10/1
  SELF_Demux/11(heading [deg]) -> Terminator_11/1
  SELF_Demux/12(yaw rate [deg/s]) -> Terminator_12/1

== system TSR_Scenario_005_cs/SELF_Actros_1860_1 ==
  Selector : Selector  IndexMode=Zero-based  IndexOptions=Starting index (dialog)  Indices=PortIndex  OutputSizes=PortWidth(1)
  U : Outport
  wires:
  Selector/1 -> U/1
  [SimCoreSensors] -> Selector/1

== system TSR_Scenario_005_cs/SELF_Actros_2541_1 ==
  Selector : Selector  IndexMode=Zero-based  IndexOptions=Starting index (dialog)  Indices=PortIndex  OutputSizes=PortWidth(1)
  U : Outport
  wires:
  Selector/1 -> U/1
  [SimCoreSensors] -> Selector/1

== system TSR_Scenario_005_cs/SELF_Audi_A8_1 ==
  Selector : Selector  IndexMode=Zero-based  IndexOptions=Starting index (dialog)  Indices=PortIndex  OutputSizes=PortWidth(1)
  U : Outport
  wires:
  Selector/1 -> U/1
  [SimCoreSensors] -> Selector/1

== system TSR_Scenario_005_cs/SELF_BMW_X5_1 ==
  Selector : Selector  IndexMode=Zero-based  IndexOptions=Starting index (dialog)  Indices=PortIndex  OutputSizes=PortWidth(1)
  U : Outport
  wires:
  Selector/1 -> U/1
  [SimCoreSensors] -> Selector/1

== system TSR_Scenario_005_cs/SELF_BMW_X5_2 ==
  Selector : Selector  IndexMode=Zero-based  IndexOptions=Starting index (dialog)  Indices=PortIndex  OutputSizes=PortWidth(1)
  U : Outport
  wires:
  Selector/1 -> U/1
  [SimCoreSensors] -> Selector/1

== system TSR_Scenario_005_cs/SELF_BMW_X5_3 ==
  Selector : Selector  IndexMode=Zero-based  IndexOptions=Starting index (dialog)  Indices=PortIndex  OutputSizes=PortWidth(1)
  U : Outport
  wires:
  Selector/1 -> U/1
  [SimCoreSensors] -> Selector/1

== system TSR_Scenario_005_cs/SELF_BMW_Z3_1 ==
  Selector : Selector  IndexMode=Zero-based  IndexOptions=Starting index (dialog)  Indices=PortIndex  OutputSizes=PortWidth(1)
  U : Outport
  wires:
  Selector/1 -> U/1
  [SimCoreSensors] -> Selector/1

== system TSR_Scenario_005_cs/SELF_CarTrailer_SingleAxle_1 ==
  Selector : Selector  IndexMode=Zero-based  IndexOptions=Starting index (dialog)  Indices=PortIndex  OutputSizes=PortWidth(1)
  U : Outport
  wires:
  Selector/1 -> U/1
  [SimCoreSensors] -> Selector/1

== system TSR_Scenario_005_cs/SELF_Citroen_C3_1 ==
  Selector : Selector  IndexMode=Zero-based  IndexOptions=Starting index (dialog)  Indices=PortIndex  OutputSizes=PortWidth(1)
  U : Outport
  wires:
  Selector/1 -> U/1
  [SimCoreSensors] -> Selector/1

== system TSR_Scenario_005_cs/SELF_Daf_95_1 ==
  Selector : Selector  IndexMode=Zero-based  IndexOptions=Starting index (dialog)  Indices=PortIndex  OutputSizes=PortWidth(1)
  U : Outport
  wires:
  Selector/1 -> U/1
  [SimCoreSensors] -> Selector/1

== system TSR_Scenario_005_cs/SELF_Female_RegularAni_1 ==
  Selector : Selector  IndexMode=Zero-based  IndexOptions=Starting index (dialog)  Indices=PortIndex  OutputSizes=PortWidth(1)
  U : Outport
  wires:
  Selector/1 -> U/1
  [SimCoreSensors] -> Selector/1
